simulink model "x64_32s_001"  (Simulink 7.4) (continued)
== system x64_32s_001 == (continued)
      mask CallbackString:
      | |||map_tail = get_param(gcb, 'map_tail');
      | set_param_state(gcb, 'LargerFFTSize', map_tail);
      | set_param_state(gcb, 'StartStage', map_tail);|||||||||fft_callback_arch(gcb);|||specify_mult = get_param(gcb, 'specify_mult');
      | set_param_state(gcb, 'mult_spec', specify_mult);||hardcode_shifts = get_param(gcb, 'hardcode_shifts');
      | set_param_state(gcb, 'shift_schedule', hardcode_shifts);||fft_callback_dsp48_adders(gcb);
      mask TabNameString: Basic,Basic,Basic,Basic,Basic,Basic,Latency,Latency,Latency,Latency,Implementation,Implementation,Implementation,Implementation,Implementation,Implementation,Implementation,Implementation,Implementation,Implementation
  fft_direct2 : Subsystem  AncestorBlock=casper_library_ffts/fft_direct  mask(fft_direct){FFTSize=3, input_bit_width=18, coeff_bit_width=18, map_tail=off, LargerFFTSize=5, StartStage=4, add_latency=2, mult_latency=2, bram_latency=2, conv_latency=2, quantization=Round  (unbiased: +/- Inf), overflow=Saturate, arch=Virtex5, opt_target=logic, coeffs_bit_limit=8, specify_mult=off, mult_spec=[2                                      2], hardcode_shifts=off, shift_schedule=[1                                      1], dsp48_adders=on}
      mask prompts: FFTSize "Size of FFT: (2^? pts)"; input_bit_width "Input/Output Bit Width"; coeff_bit_width "Coefficient Bit Width"; map_tail "Map Tail of Larger FFT"; LargerFFTSize "Size of Larger FFT (2^?)"; StartStage "Start Stage of Map Tail"; add_latency "Add Latency"; mult_latency "Mult Latency"; bram_latency "BRAM Latency"; conv_latency "Convert latency"; quantization "Quantization Behavior"; overflow "Overflow Behavior"; arch "Architecture"; opt_target "Use less"; coeffs_bit_limit "Number bits above which to store stage's coefficients in BRAM (2^? bits)"; specify_mult "Specify multiplier use (behavioural HDL if not specified)"; mult_spec "Multiplier specification (0=core, 1=behavioural HDL, 2=embedded) (left=1st stage)"; hardcode_shifts "Hardcode shift schedule"; shift_schedule "Shift schedule"; dsp48_adders "DSP48 adders in butterfly"
      mask help:
      | FFT internal block.
      |
      | Implements an N-point FFT as the classic butterfly structure,
      | with N inputs and N outputs.
      mask init: same as x64_32s_001/fft_direct1
      settings: AttributesFormatString=|
      | Virtex5
      | stages [4  5  6] of 3
      | [18,18]
      | Round  (unbiased: +/- Inf)
      | Saturate
      mask StyleString: edit,edit,edit,checkbox,edit,edit,edit,edit,edit,edit,popup(Truncate|Round  (unbiased: +/- Inf)|Round  (unbiased: Even Values)),popup(Wrap|Saturate|Error),popup(Virtex2Pro|Virtex5),popup(logic|multipliers),edit,checkbox,edit,checkbox,edit,checkbox
      mask CallbackString:
      | |||map_tail = get_param(gcb, 'map_tail');
      | set_param_state(gcb, 'LargerFFTSize', map_tail);
      | set_param_state(gcb, 'StartStage', map_tail);|||||||||fft_callback_arch(gcb);|||specify_mult = get_param(gcb, 'specify_mult');
      | set_param_state(gcb, 'mult_spec', specify_mult);||hardcode_shifts = get_param(gcb, 'hardcode_shifts');
      | set_param_state(gcb, 'shift_schedule', hardcode_shifts);||fft_callback_dsp48_adders(gcb);
      mask TabNameString: Basic,Basic,Basic,Basic,Basic,Basic,Latency,Latency,Latency,Latency,Implementation,Implementation,Implementation,Implementation,Implementation,Implementation,Implementation,Implementation,Implementation,Implementation
  inst_seng_id0 : Subsystem  Tag=xps:sw_reg  mask{io_dir=To Processor, arith_type=Unsigned, bitwidth=32, bin_pt=0, sample_period=1, gw_name=x64_32s_001_inst_seng_id0_user_data_in}
      mask prompts: io_dir "I/O direction"; arith_type "Data Type"; bitwidth "Data bitwidth"; bin_pt "Data binary point"; sample_period "Sample period"; gw_name "Mask Parameter 6:"
      mask init:
      | register_mask;
      mask StyleString: popup(From Processor|To Processor),popup(Unsigned|Signed  (2's comp)),edit,edit,edit,edit
  mcnt_reg1 : xbsIndex_r4/Register  init=0  rst=off  en=on  dbl_ovrd=off  block_type=register
      settings: block_version=11.4
  mini_vacc : Subsystem
  mini_vacc_mux1 : Subsystem  mask{vec_len=3}
      mask prompts: vec_len "vector length (2^?)"
  payload_cnt1 : S-Function  LoadFcn=xlBlockLoadCallback(gcbh);  FunctionName=sysgen  Parameters=ptable_  EnableBusSupport=off  mask(Xilinx Counter Block){infoedit=Hardware notes: Free running counters are the least expensive in hardware.  A count limited counter is implemented by combining a counter with a comparator., cnt_type_sgadvanced=, cnt_type=Free Running, cnt_to=0, operation_sgadvanced=, operation=Up, start_count=0, cnt_by_val=1, arith_type_sgadvanced=, arith_type=Unsigned, n_bits=32, bin_pt=0, load_pin_sgadvanced=, load_pin=off, rst_sgadvanced=, rst=on, en_sgadvanced=, en=on, explicit_period_sgadvanced=, explicit_period=off, period=1, dbl_ovrd_sgadvanced=, dbl_ovrd=off, use_behavioral_HDL_sgadvanced=, use_behavioral_HDL=off, implementation_sgadvanced=, implementation=Fabric, xl_use_area_sgadvanced=, xl_use_area=off, xl_area=[17 32 0 32 0 0 0], use_rpm=on, xlcconfig=, has_advanced_control=0, sggui_pos=20,20,348,618, block_type=counter, block_version=10.1.3, sg_icon_stat=30,35,2,1,white,blue,0,d605779c,right,, sg_mask_display=|, sg_list_contents=, sg_blockgui_xml=}
      mask sg_mask_display=|
      | fprintf('','COMMENT: begin icon graphics');
      | patch([0 1 1 0 ],[0 0 1 1 ],[0.77 0.82 0.91]);
      | patch([0.233333 0.0666667 0.3 0.0666667 0.233333 0.5 0.566667 0.633333 0.9 0.666667 0.466667 0.3 0.5 0.3 0.466667 0.666667 0.9 0.633333 0.566667 0.5 0.233333 ],[0.171429 0.314286 0.514286 0.714286 0.857143 0.857143 0.8 0.857143 0.857143 0.657143 0.828571 0.685714 0.514286 0.342857 0.2 0.371429 0.171429 0.171429 0.228571 0.171429 0.171429 ],[0.98 0.96 0.92]);
      | plot([0 1 1 0 0 ],[0 0 1 1 0 ]);
      | fprintf('','COMMENT: end icon graphics');
      | fprintf('','COMMENT: begin icon text');
      | color('black');port_label('input',1,'rst');
      | color('black');port_label('input',2,'en');
      | color('black');port_label('output',1,'out');
      | fprintf('','COMMENT: end icon text');
      mask prompts: infoedit ""; cnt_type_sgadvanced ""; cnt_type "Counter type"; cnt_to "Count to value"; operation_sgadvanced ""; operation "Count direction"; start_count "Initial value"; cnt_by_val "Step"; arith_type_sgadvanced ""; arith_type "Output type"; n_bits "Number of bits"; bin_pt "Binary point"; load_pin_sgadvanced ""; load_pin "Provide load port"; rst_sgadvanced ""; rst "Provide synchronous reset port"; en_sgadvanced ""; en "Provide enable port"; explicit_period_sgadvanced ""; explicit_period "Sample period source"; period "Explicit period"; dbl_ovrd_sgadvanced ""; dbl_ovrd "Override with doubles"; use_behavioral_HDL_sgadvanced ""; use_behavioral_HDL "Use behavioral HDL (otherwise use core)"; implementation_sgadvanced ""; implementation "Implement using"; xl_use_area_sgadvanced ""; xl_use_area "Define FPGA area for resource estimation"; xl_area "FPGA area [slices, FFs, BRAMs, LUTs, IOBs, emb. mults, TBUFs]"; use_rpm ""; xlcconfig ""; has_advanced_control ""; sggui_pos ""; block_type ""; block_version ""; sg_icon_stat ""; sg_mask_display ""; sg_list_contents ""; sg_blockgui_xml ""
      mask help: Hardware notes: Free running counters are the least expensive in hardware. A count limited counter is implemented by combining a counter with a comparator.
      mask init:
      | try
      |   tmp_gcb = gcb;
      |   tmp_gcbh = gcbh;
      |   if (strcmp('SysGenIndex',get_param(bdroot(tmp_gcbh),'tag')) && ~isempty(regexp(bdroot(tmp_gcb), '^xbs', 'once')))
      |     return;
      |   end;
      |   xlMungeMaskParams;
      |   block_config = 'sysgen_blockset:Counter_config';
      | 
      |   block_type='counter';
      | 
      |   serialized_declarations = '{''arith_type''=>''Int'',''bin_pt''=>''Int'',''cnt_by_val''=>''Double'',''cnt_to''=>''Double'',''cnt_type''=>''Int'',''en''=>''Int'',''explicit_period''=>''Int'',''implementation''=>''String'',''load_pin''=>''Int'',''n_bits''=>''Int'',''operation''=>''Int'',''period''=>''Double'',''rst''=>''Int'',''start_count''=>''Double'',''use_behavioral_HDL''=>''Int'',''use_rpm''=>''String'',''xlcconfig''=>''String'',''block_type''=>''String''}';
      |   ptable_ = xlblockprep(get_param(tmp_gcb, 'MaskWSVariables'));
      | catch
      |   global dbgsysgen;
      |   if(~isempty(dbgsysgen) && dbgsysgen)
      |     e = regexprep(lasterr, '\n', '\nError: ');
      |     disp(['Error: While running MaskInit code on block ' tmp_gcb ': ' e]);
      |     error(e);
      |   end
      | end
      settings: CopyFcn=xlBlockMoveCallback(gcbh);  DeleteFcn=xlDestroyGui(gcbh);  ModelCloseFcn=xlDestroyGui(gcbh);  PreSaveFcn=xlBlockPreSaveCallback(gcbh);  PostSaveFcn=xlBlockPostSaveCallback(gcbh);  DestroyFcn=xlDestroyGui(gcbh);  OpenFcn=xlOpenGui(gcbh, 'counter_gui.xml', @counterenablement, -1)  CloseFcn=xlDestroyGui(gcbh);  MoveFcn=xlBlockMoveCallback(gcbh);
      mask Help: eval('');xlDoc('-book','sysgen','-topic','Counter');
      mask StyleString: edit,edit,popup(Free Running|Count Limited),edit,edit,popup(Up|Down|Up/Down),edit,edit,edit,popup(Signed  (2's comp)|Unsigned),edit,edit,edit,checkbox,edit,checkbox,edit,checkbox,edit,popup(on|off),edit,edit,checkbox,edit,checkbox,edit,popup(Fabric|DSP48),edit,checkbox,edit,edit,edit,edit,edit,edit,edit,edit,edit,edit,edit
      mask Display:
      | fprintf('','COMMENT: begin icon graphics');
      | patch([0 1 1 0 ],[0 0 1 1 ],[0.77 0.82 0.91]);
      | patch([0.233333 0.0666667 0.3 0.0666667 0.233333 0.5 0.566667 0.633333 0.9 0.666667 0.466667 0.3 0.5 0.3 0.466667 0.666667 0.9 0.633333 0.566667 0.5 0.233333 ],[0.171429 0.314286 0.514286 0.714286 0.857143 0.857143 0.8 0.857143 0.857143 0.657143 0.828571 0.685714 0.514286 0.342857 0.2 0.371429 0.171429 0.171429 0.228571 0.171429 0.171429 ],[0.98 0.96 0.92]);
      | plot([0 1 1 0 0 ],[0 0 1 1 0 ]);
      | fprintf('','COMMENT: end icon graphics');
      | fprintf('','COMMENT: begin icon text');
      | color('black');port_label('input',1,'rst');
      | color('black');port_label('input',2,'en');
      | color('black');port_label('output',1,'out');
      | fprintf('','COMMENT: end icon text');
  payload_cnt2 : S-Function  LoadFcn=xlBlockLoadCallback(gcbh);  FunctionName=sysgen  Parameters=ptable_  EnableBusSupport=off  mask(Xilinx Counter Block){infoedit=Hardware notes: Free running counters are the least expensive in hardware.  A count limited counter is implemented by combining a counter with a comparator., cnt_type_sgadvanced=, cnt_type=Free Running, cnt_to=0, operation_sgadvanced=, operation=Up, start_count=0, cnt_by_val=1, arith_type_sgadvanced=, arith_type=Unsigned, n_bits=32, bin_pt=0, load_pin_sgadvanced=, load_pin=off, rst_sgadvanced=, rst=on, en_sgadvanced=, en=on, explicit_period_sgadvanced=, explicit_period=off, period=1, dbl_ovrd_sgadvanced=, dbl_ovrd=off, use_behavioral_HDL_sgadvanced=, use_behavioral_HDL=off, implementation_sgadvanced=, implementation=Fabric, xl_use_area_sgadvanced=, xl_use_area=off, xl_area=[17 32 0 32 0 0 0], use_rpm=on, xlcconfig=, has_advanced_control=0, sggui_pos=20,20,348,618, block_type=counter, block_version=10.1.3, sg_icon_stat=30,35,2,1,white,blue,0,d605779c,right,, sg_mask_display=|, sg_list_contents=, sg_blockgui_xml=}
      mask sg_mask_display=|
      | fprintf('','COMMENT: begin icon graphics');
      | patch([0 1 1 0 ],[0 0 1 1 ],[0.77 0.82 0.91]);
      | patch([0.233333 0.0666667 0.3 0.0666667 0.233333 0.5 0.566667 0.633333 0.9 0.666667 0.466667 0.3 0.5 0.3 0.466667 0.666667 0.9 0.633333 0.566667 0.5 0.233333 ],[0.171429 0.314286 0.514286 0.714286 0.857143 0.857143 0.8 0.857143 0.857143 0.657143 0.828571 0.685714 0.514286 0.342857 0.2 0.371429 0.171429 0.171429 0.228571 0.171429 0.171429 ],[0.98 0.96 0.92]);
      | plot([0 1 1 0 0 ],[0 0 1 1 0 ]);
      | fprintf('','COMMENT: end icon graphics');
      | fprintf('','COMMENT: begin icon text');
      | color('black');port_label('input',1,'rst');
      | color('black');port_label('input',2,'en');
      | color('black');port_label('output',1,'out');
      | fprintf('','COMMENT: end icon text');
      mask prompts: infoedit ""; cnt_type_sgadvanced ""; cnt_type "Counter type"; cnt_to "Count to value"; operation_sgadvanced ""; operation "Count direction"; start_count "Initial value"; cnt_by_val "Step"; arith_type_sgadvanced ""; arith_type "Output type"; n_bits "Number of bits"; bin_pt "Binary point"; load_pin_sgadvanced ""; load_pin "Provide load port"; rst_sgadvanced ""; rst "Provide synchronous reset port"; en_sgadvanced ""; en "Provide enable port"; explicit_period_sgadvanced ""; explicit_period "Sample period source"; period "Explicit period"; dbl_ovrd_sgadvanced ""; dbl_ovrd "Override with doubles"; use_behavioral_HDL_sgadvanced ""; use_behavioral_HDL "Use behavioral HDL (otherwise use core)"; implementation_sgadvanced ""; implementation "Implement using"; xl_use_area_sgadvanced ""; xl_use_area "Define FPGA area for resource estimation"; xl_area "FPGA area [slices, FFs, BRAMs, LUTs, IOBs, emb. mults, TBUFs]"; use_rpm ""; xlcconfig ""; has_advanced_control ""; sggui_pos ""; block_type ""; block_version ""; sg_icon_stat ""; sg_mask_display ""; sg_list_contents ""; sg_blockgui_xml ""
      mask help: Hardware notes: Free running counters are the least expensive in hardware. A count limited counter is implemented by combining a counter with a comparator.
      mask init: same as x64_32s_001/payload_cnt1
      settings: CopyFcn=xlBlockMoveCallback(gcbh);  DeleteFcn=xlDestroyGui(gcbh);  ModelCloseFcn=xlDestroyGui(gcbh);  PreSaveFcn=xlBlockPreSaveCallback(gcbh);  PostSaveFcn=xlBlockPostSaveCallback(gcbh);  DestroyFcn=xlDestroyGui(gcbh);  OpenFcn=xlOpenGui(gcbh, 'counter_gui.xml', @counterenablement, -1)  CloseFcn=xlDestroyGui(gcbh);  MoveFcn=xlBlockMoveCallback(gcbh);
      mask Help: eval('');xlDoc('-book','sysgen','-topic','Counter');
      mask StyleString: edit,edit,popup(Free Running|Count Limited),edit,edit,popup(Up|Down|Up/Down),edit,edit,edit,popup(Signed  (2's comp)|Unsigned),edit,edit,edit,checkbox,edit,checkbox,edit,checkbox,edit,popup(on|off),edit,edit,checkbox,edit,checkbox,edit,popup(Fabric|DSP48),edit,checkbox,edit,edit,edit,edit,edit,edit,edit,edit,edit,edit,edit
      mask Display:
      | fprintf('','COMMENT: begin icon graphics');
      | patch([0 1 1 0 ],[0 0 1 1 ],[0.77 0.82 0.91]);
      | patch([0.233333 0.0666667 0.3 0.0666667 0.233333 0.5 0.566667 0.633333 0.9 0.666667 0.466667 0.3 0.5 0.3 0.466667 0.666667 0.9 0.633333 0.566667 0.5 0.233333 ],[0.171429 0.314286 0.514286 0.714286 0.857143 0.857143 0.8 0.857143 0.857143 0.657143 0.828571 0.685714 0.514286 0.342857 0.2 0.371429 0.171429 0.171429 0.228571 0.171429 0.171429 ],[0.98 0.96 0.92]);
      | plot([0 1 1 0 0 ],[0 0 1 1 0 ]);
      | fprintf('','COMMENT: end icon graphics');
      | fprintf('','COMMENT: begin icon text');
      | color('black');port_label('input',1,'rst');
      | color('black');port_label('input',2,'en');
      | color('black');port_label('output',1,'out');
      | fprintf('','COMMENT: end icon text');
  payload_cnt3 : S-Function  LoadFcn=xlBlockLoadCallback(gcbh);  FunctionName=sysgen  Parameters=ptable_  EnableBusSupport=off  mask(Xilinx Counter Block){infoedit=Hardware notes: Free running counters are the least expensive in hardware.  A count limited counter is implemented by combining a counter with a comparator., cnt_type_sgadvanced=, cnt_type=Free Running, cnt_to=0, operation_sgadvanced=, operation=Up, start_count=0, cnt_by_val=1, arith_type_sgadvanced=, arith_type=Unsigned, n_bits=32, bin_pt=0, load_pin_sgadvanced=, load_pin=off, rst_sgadvanced=, rst=on, en_sgadvanced=, en=on, explicit_period_sgadvanced=, explicit_period=off, period=1, dbl_ovrd_sgadvanced=, dbl_ovrd=off, use_behavioral_HDL_sgadvanced=, use_behavioral_HDL=off, implementation_sgadvanced=, implementation=Fabric, xl_use_area_sgadvanced=, xl_use_area=off, xl_area=[17 32 0 32 0 0 0], use_rpm=on, xlcconfig=, has_advanced_control=0, sggui_pos=20,20,348,618, block_type=counter, block_version=10.1.3, sg_icon_stat=30,35,2,1,white,blue,0,d605779c,right,, sg_mask_display=|, sg_list_contents=, sg_blockgui_xml=}
      mask sg_mask_display=|
      | fprintf('','COMMENT: begin icon graphics');
      | patch([0 1 1 0 ],[0 0 1 1 ],[0.77 0.82 0.91]);
      | patch([0.233333 0.0666667 0.3 0.0666667 0.233333 0.5 0.566667 0.633333 0.9 0.666667 0.466667 0.3 0.5 0.3 0.466667 0.666667 0.9 0.633333 0.566667 0.5 0.233333 ],[0.171429 0.314286 0.514286 0.714286 0.857143 0.857143 0.8 0.857143 0.857143 0.657143 0.828571 0.685714 0.514286 0.342857 0.2 0.371429 0.171429 0.171429 0.228571 0.171429 0.171429 ],[0.98 0.96 0.92]);
      | plot([0 1 1 0 0 ],[0 0 1 1 0 ]);
      | fprintf('','COMMENT: end icon graphics');
      | fprintf('','COMMENT: begin icon text');
      | color('black');port_label('input',1,'rst');
      | color('black');port_label('input',2,'en');
      | color('black');port_label('output',1,'out');
      | fprintf('','COMMENT: end icon text');
      mask prompts: infoedit ""; cnt_type_sgadvanced ""; cnt_type "Counter type"; cnt_to "Count to value"; operation_sgadvanced ""; operation "Count direction"; start_count "Initial value"; cnt_by_val "Step"; arith_type_sgadvanced ""; arith_type "Output type"; n_bits "Number of bits"; bin_pt "Binary point"; load_pin_sgadvanced ""; load_pin "Provide load port"; rst_sgadvanced ""; rst "Provide synchronous reset port"; en_sgadvanced ""; en "Provide enable port"; explicit_period_sgadvanced ""; explicit_period "Sample period source"; period "Explicit period"; dbl_ovrd_sgadvanced ""; dbl_ovrd "Override with doubles"; use_behavioral_HDL_sgadvanced ""; use_behavioral_HDL "Use behavioral HDL (otherwise use core)"; implementation_sgadvanced ""; implementation "Implement using"; xl_use_area_sgadvanced ""; xl_use_area "Define FPGA area for resource estimation"; xl_area "FPGA area [slices, FFs, BRAMs, LUTs, IOBs, emb. mults, TBUFs]"; use_rpm ""; xlcconfig ""; has_advanced_control ""; sggui_pos ""; block_type ""; block_version ""; sg_icon_stat ""; sg_mask_display ""; sg_list_contents ""; sg_blockgui_xml ""
      mask help: Hardware notes: Free running counters are the least expensive in hardware. A count limited counter is implemented by combining a counter with a comparator.
      mask init: same as x64_32s_001/payload_cnt1
      settings: CopyFcn=xlBlockMoveCallback(gcbh);  DeleteFcn=xlDestroyGui(gcbh);  ModelCloseFcn=xlDestroyGui(gcbh);  PreSaveFcn=xlBlockPreSaveCallback(gcbh);  PostSaveFcn=xlBlockPostSaveCallback(gcbh);  DestroyFcn=xlDestroyGui(gcbh);  OpenFcn=xlOpenGui(gcbh, 'counter_gui.xml', @counterenablement, -1)  CloseFcn=xlDestroyGui(gcbh);  MoveFcn=xlBlockMoveCallback(gcbh);
      mask Help: eval('');xlDoc('-book','sysgen','-topic','Counter');
      mask StyleString: edit,edit,popup(Free Running|Count Limited),edit,edit,popup(Up|Down|Up/Down),edit,edit,edit,popup(Signed  (2's comp)|Unsigned),edit,edit,edit,checkbox,edit,checkbox,edit,checkbox,edit,popup(on|off),edit,edit,checkbox,edit,checkbox,edit,popup(Fabric|DSP48),edit,checkbox,edit,edit,edit,edit,edit,edit,edit,edit,edit,edit,edit
      mask Display:
      | fprintf('','COMMENT: begin icon graphics');
      | patch([0 1 1 0 ],[0 0 1 1 ],[0.77 0.82 0.91]);
      | patch([0.233333 0.0666667 0.3 0.0666667 0.233333 0.5 0.566667 0.633333 0.9 0.666667 0.466667 0.3 0.5 0.3 0.466667 0.666667 0.9 0.633333 0.566667 0.5 0.233333 ],[0.171429 0.314286 0.514286 0.714286 0.857143 0.857143 0.8 0.857143 0.857143 0.657143 0.828571 0.685714 0.514286 0.342857 0.2 0.371429 0.171429 0.171429 0.228571 0.171429 0.171429 ],[0.98 0.96 0.92]);
      | plot([0 1 1 0 0 ],[0 0 1 1 0 ]);
      | fprintf('','COMMENT: end icon graphics');
      | fprintf('','COMMENT: begin icon text');
      | color('black');port_label('input',1,'rst');
      | color('black');port_label('input',2,'en');
      | color('black');port_label('output',1,'out');
      | fprintf('','COMMENT: end icon text');
  pow_sim_mux : Subsystem
  pow_sim_mux1 : Subsystem
  pow_sim_mux10 : Subsystem
  pow_sim_mux11 : Subsystem
  pow_sim_mux12 : Subsystem
  pow_sim_mux13 : Subsystem
  pow_sim_mux14 : Subsystem
  pow_sim_mux15 : Subsystem
  pow_sim_mux2 : Subsystem
  pow_sim_mux3 : Subsystem
  pow_sim_mux4 : Subsystem
  pow_sim_mux5 : Subsystem
  pow_sim_mux6 : Subsystem
  pow_sim_mux7 : Subsystem
  pow_sim_mux8 : Subsystem
  pow_sim_mux9 : Subsystem
  qdr_in_cnt : Subsystem  Tag=xps:sw_reg  mask{io_dir=To Processor, arith_type=Unsigned, bitwidth=32, bin_pt=0, sample_period=1, gw_name=x64_32s_001_qdr_in_cnt_user_data_in}
      mask prompts: io_dir "I/O direction"; arith_type "Data Type"; bitwidth "Data bitwidth"; bin_pt "Data binary point"; sample_period "Sample period"; gw_name "Mask Parameter 6:"
      mask init:
      | register_mask;
      mask StyleString: popup(From Processor|To Processor),popup(Unsigned|Signed  (2's comp)),edit,edit,edit,edit
  qdr_vacc : Subsystem  AncestorBlock=casper_library_accumulators/qdr_vacc  mask{vector_len=1024*32*4}
      mask prompts: vector_len "Vector length??(# cycles)"
      mask help:
      | A vector accumulator for medium length vectors designed for ROACH's QDR memory.
      |
      | Originally designed for the packetised correlator, this block accepts input data of type Fix_32_0. OOB_data_in provides an extra 4 bits that are accumulated seperately (originally designed to keep track of how many "bad" values in a vector were accumulated).
      |
      | Minimum vector length is 2 * burst_length.
      | Minimum burst length is 10 (recommended default is 2^4=16).
      |
      | The vector length does not need to be a multiple of the burst length.
      mask init:
      | vector_bits = ceil(log2(vector_len));
      | burst_bits = 4;
      | burst_len = 2^burst_bits;
      | 
      | fmtstr = sprintf('Vector Length: %d', vector_len);
      | set_param(gcb, 'AttributesFormatString', fmtstr);
      settings: AttributesFormatString=Vector Length: 131072
  snap_vacc0 : Subsystem  AncestorBlock=casper_library/Scopes/snap  mask(snap){nsamples=11}
      mask prompts: nsamples "No. of samples(2^?)"
      mask help:
      | The <i>snap</i> block provides a packaged solution to capturing data from
      | the FPGA fabric and making it accessible from the CPU.  The block comes in
      | 2 flavors: <i>snap</i> (which captures to a 32 bit wide Shared BRAM), and
      | <i>snap64</i> (which captures to 2x32 bit wide Shared BRAMs to effect a
      | 64 bit capture).
      mask Help: eval('xlWeb([getenv(''MLIB_ROOT''), ''\doc\htmlblocks\sys_snap\sys_snap.html''])')
  square_transposer : Subsystem  AncestorBlock=casper_library/Reorder/square_transposer  mask(square_transposer){n_inputs=3}
      mask prompts: n_inputs "Number of inputs (2^?):"
      mask init:
      | square_transposer_init(gcb,...
      |     'n_inputs', n_inputs);
      settings: AttributesFormatString=n_inputs=3
      mask Help: eval('xlWeb(''http://casper.berkeley.edu/wiki/Square_transposer'')')
  status : Subsystem  Tag=xps:sw_reg  mask{io_dir=To Processor, arith_type=Unsigned, bitwidth=32, bin_pt=0, sample_period=1, gw_name=x64_32s_001_status_user_data_in}
      mask prompts: io_dir "I/O direction"; arith_type "Data Type"; bitwidth "Data bitwidth"; bin_pt "Data binary point"; sample_period "Sample period"; gw_name "Mask Parameter 6:"
      mask init:
      | register_mask;
      mask StyleString: popup(From Processor|To Processor),popup(Unsigned|Signed  (2's comp)),edit,edit,edit,edit
  sync_gen : Subsystem  mask{nChans=10, nXeng=0}
      mask prompts: nChans "Number of channels (total) (2^?)"; nXeng "Number of X engines (2^?)"
  uncram1 : Subsystem  AncestorBlock=gavrt_library/uncram  mask(uncram){num_slice=4, slice_width=8, bin_pt=0, arith_type=0}
      mask prompts: num_slice "Number of slices"; slice_width "Slice Width"; bin_pt "Output Binary Point"; arith_type "Output Arithmetic Type (0=Unsigned, 1=Signed)"
      mask help: Takes a concatenated input and slices it up into even pieces and reinterprets them as signed fixed point numbers with a given binary point.
      mask init:
      | uncram_init(gcb, ...
      |     'num_slice', num_slice, ...
      |     'slice_width', slice_width, ...
      |     'bin_pt', bin_pt, ...
      |     'arith_type', arith_type);
      settings: AttributesFormatString=UFix_8_0
  vacc_cnt0 : Subsystem  Tag=xps:sw_reg  mask{io_dir=To Processor, arith_type=Unsigned, bitwidth=32, bin_pt=0, sample_period=1, latency=0}
      mask prompts: io_dir "I/O direction"; arith_type "Data Type"; bitwidth "Data bitwidth"; bin_pt "Data binary point"; sample_period "Sample period"
      mask init:
      | gw_name = clear_name(gcb);
      | config.source = str2func('software_register_init');
      | config.toplevel = gcb;
      | xBlock( config, {io_dir, arith_type, bitwidth, bin_pt, ... 
      |     sample_period, gw_name, latency});
      mask StyleString: popup(From Processor|To Processor),popup(Unsigned|Signed  (2's comp)),edit,edit,edit,edit
  vacc_err_cnt0 : Subsystem  Tag=xps:sw_reg  mask{io_dir=To Processor, arith_type=Unsigned, bitwidth=32, bin_pt=0, sample_period=1, latency=0}
      mask prompts: io_dir "I/O direction"; arith_type "Data Type"; bitwidth "Data bitwidth"; bin_pt "Data binary point"; sample_period "Sample period"
      mask init:
      | gw_name = clear_name(gcb);
      | config.source = str2func('software_register_init');
      | config.toplevel = gcb;
      | xBlock( config, {io_dir, arith_type, bitwidth, bin_pt, ... 
      |     sample_period, gw_name, latency});
      mask StyleString: popup(From Processor|To Processor),popup(Unsigned|Signed  (2's comp)),edit,edit,edit,edit
  vacc_mcnt_h0 : Subsystem  Tag=xps:sw_reg  mask{io_dir=To Processor, arith_type=Unsigned, bitwidth=32, bin_pt=0, sample_period=1, latency=0}
      mask prompts: io_dir "I/O direction"; arith_type "Data Type"; bitwidth "Data bitwidth"; bin_pt "Data binary point"; sample_period "Sample period"
      mask init:
      | gw_name = clear_name(gcb);
      | config.source = str2func('software_register_init');
      | config.toplevel = gcb;
      | xBlock( config, {io_dir, arith_type, bitwidth, bin_pt, ... 
      |     sample_period, gw_name, latency});
      mask StyleString: popup(From Processor|To Processor),popup(Unsigned|Signed  (2's comp)),edit,edit,edit,edit
  vacc_mcnt_l0 : Subsystem  Tag=xps:sw_reg  mask{io_dir=To Processor, arith_type=Unsigned, bitwidth=32, bin_pt=0, sample_period=1, latency=0}
      mask prompts: io_dir "I/O direction"; arith_type "Data Type"; bitwidth "Data bitwidth"; bin_pt "Data binary point"; sample_period "Sample period"
      mask init:
      | gw_name = clear_name(gcb);
      | config.source = str2func('software_register_init');
      | config.toplevel = gcb;
      | xBlock( config, {io_dir, arith_type, bitwidth, bin_pt, ... 
      |     sample_period, gw_name, latency});
      mask StyleString: popup(From Processor|To Processor),popup(Unsigned|Signed  (2's comp)),edit,edit,edit,edit
  vacc_monitor : Subsystem
  vacc_monitor1 : Subsystem
  vacc_monitor2 : Subsystem
  vacc_sim_mux : Subsystem
  x_window : Subsystem
  xaui_TVG : Subsystem
  xaui_buf0 : Subsystem
  xaui_cnt0 : Subsystem  Tag=xps:sw_reg  mask{io_dir=To Processor, arith_type=Unsigned, bitwidth=32, bin_pt=0, sample_period=1, gw_name=x64_32s_001_xaui_cnt0_user_data_in}
      mask prompts: io_dir "I/O direction"; arith_type "Data Type"; bitwidth "Data bitwidth"; bin_pt "Data binary point"; sample_period "Sample period"; gw_name "Mask Parameter 6:"
      mask init:
      | register_mask;
      mask StyleString: popup(From Processor|To Processor),popup(Unsigned|Signed  (2's comp)),edit,edit,edit,edit
  xaui_error_cnt0 : Subsystem  Tag=xps:sw_reg  mask{io_dir=To Processor, arith_type=Unsigned, bitwidth=32, bin_pt=0, sample_period=1, gw_name=x64_32s_001_xaui_error_cnt0_user_data_in}
      mask prompts: io_dir "I/O direction"; arith_type "Data Type"; bitwidth "Data bitwidth"; bin_pt "Data binary point"; sample_period "Sample period"; gw_name "Mask Parameter 6:"
      mask init:
      | register_mask;
      mask StyleString: popup(From Processor|To Processor),popup(Unsigned|Signed  (2's comp)),edit,edit,edit,edit
  xaui_pack0 : Subsystem  mask{payload_len=2^5, xeng_bits=0, skip_xeng_bits=0, skip_ant_bits=4}
      mask prompts: payload_len "Payload Length (in 64 bit words)"; xeng_bits "Number of X engines (2^?)"; skip_xeng_bits "X engines per IP address (2^?)"; skip_ant_bits "Antennas per XAUI link (2^?)"
      mask help: Packetizes data coming in over a XAUI interface. A packet consists of a 64 bit header (48 bits of "mcnt" and 16 bits of antenna), followed by 64 x "payload" bits of payload. "Mcnt" (master count) is a counter which keeps track of how many packets have been transmitted since the last "mrst".
      mask init:
      | packet_len = payload_len + 1;
      | packet_bits = ceil(log2(packet_len));
      | payload_bits = ceil(log2(payload_len));
  xaui_sim_mux2 : Subsystem  mask{xaui_lat=2, start_val=1}
      mask prompts: xaui_lat "simulated XAUI latency"; start_val "count start value"
  xauibuf_out_cnt : Subsystem  Tag=xps:sw_reg  mask{io_dir=To Processor, arith_type=Unsigned, bitwidth=32, bin_pt=0, sample_period=1, gw_name=x64_32s_001_xauibuf_out_cnt_user_data_in}
      mask prompts: io_dir "I/O direction"; arith_type "Data Type"; bitwidth "Data bitwidth"; bin_pt "Data binary point"; sample_period "Sample period"; gw_name "Mask Parameter 6:"
      mask init:
      | register_mask;
      mask StyleString: popup(From Processor|To Processor),popup(Unsigned|Signed  (2's comp)),edit,edit,edit,edit
  xauibuf_window_cnt : Subsystem  Tag=xps:sw_reg  mask{io_dir=To Processor, arith_type=Unsigned, bitwidth=32, bin_pt=0, sample_period=1, gw_name=x64_32s_001_xauibuf_window_cnt_user_data_in}
      mask prompts: io_dir "I/O direction"; arith_type "Data Type"; bitwidth "Data bitwidth"; bin_pt "Data binary point"; sample_period "Sample period"; gw_name "Mask Parameter 6:"
      mask init:
      | register_mask;
      mask StyleString: popup(From Processor|To Processor),popup(Unsigned|Signed  (2's comp)),edit,edit,edit,edit
  y_window : Subsystem
  zero_mask : Subsystem
  zero_mask1 : Subsystem
  wires:
  Constant7/1 -> XAUI/1
  [xaui_rcv_rst] -> XAUI/2
  XAUI/1 -> xaui_sim_mux2/1(rx_data)
  XAUI/2 -> xaui_sim_mux2/2(rx_oob)
  XAUI/3 -> xaui_sim_mux2/3(rx_empty)
  XAUI/4 -> xaui_sim_mux2/4(rx_valid)
  XAUI/5 -> xaui_sim_mux2/5(rx_linkdown)
  XAUI/6 -> xaui_sim_mux2/6(tx_full)
  XAUI/7 -> xaui_sim_mux2/7(rx_almost_full)
  xaui_sim_mux2/7(af) -> Terminator21/1
  Constant20/1 -> ctrl_sw/1(sim_reg_in)
  ctrl_sw/1(reg_in) -> Slice/1, Slice1/1, Slice4/1, Slice5/1, Slice7/1, Slice10/1, Slice3/1, Slice2/1, Slice6/1
  Slice/1 -> [x_fft_shift]
  Constant1/1 -> XAUI/3
  Constant2/1 -> XAUI/4
  Constant3/1 -> XAUI/5
  [data_buf] -> uncram1/1(In)
  [x_fft_shift] -> fft_direct2/2(shift)
  Constant4/1 -> fft_direct2/7(in4), fft_direct2/8(in5), fft_direct2/9(in6), fft_direct2/10(in7)
  fft_direct2/1(sync_out) -> [x_fft_sync]
  fft_direct2/2(out0) -> [x0]
  fft_direct2/3(out1) -> [x1]
  fft_direct2/4(out2) -> [x2]
  fft_direct2/5(out3) -> [x3]
  fft_direct2/6(out4) -> [x4]
  fft_direct2/7(out5) -> [x5]
  fft_direct2/8(out6) -> [x6]
  fft_direct2/9(out7) -> [x7]
  fft_direct2/10(of) -> [x_fft_of]
  fft_direct1/1(sync_out) -> [y_fft_sync]
  fft_direct1/18(of) -> [y_fft_of]
  [acc_len] -> qdr_vacc/1(n_acum)
  Constant6/1 -> qdr_vacc/4(oob_data_in)
  [qdr_in_vld] -> qdr_vacc/5(we)
  [qdr_vacc_data] -> Convert3/1
  qdr_vacc/1(new_acc (ASYNC)) -> [new_acc]
  [qdr_sync] -> qdr_vacc/2(sync)
  Slice1/1 -> [y_fft_shift]
  acc_len/1(reg_in) -> [acc_len]
  Constant8/1 -> acc_len/1(sim_reg_in)
  Slice2/1 -> [xaui_rcv_rst]
  Slice3/1 -> [acc_ctr_rst]
  [x_fft_sync] -> square_transposer/1(sync)
  [x0] -> square_transposer/2(In1)
  [x1] -> square_transposer/3(In2)
  [x2] -> square_transposer/4(In3)
  [x3] -> square_transposer/5(In4)
  [x4] -> square_transposer/6(In5)
  [x5] -> square_transposer/7(In6)
  [x6] -> square_transposer/8(In7)
  [x7] -> square_transposer/9(In8)
  square_transposer/1(sync_out) -> [ct_sync_out]
  square_transposer/2(Out1) -> [y0]
  square_transposer/3(Out2) -> [y1]
  square_transposer/4(Out3) -> [y2]
  square_transposer/5(Out4) -> [y3]
  square_transposer/6(Out5) -> [y4]
  square_transposer/7(Out6) -> [y5]
  square_transposer/8(Out7) -> [y6]
  square_transposer/9(Out8) -> [y7]
  Constant9/1 -> fft_direct1/12(in9), fft_direct1/11(in8), fft_direct1/13(in10), fft_direct1/14(in11), fft_direct1/15(in12), fft_direct1/16(in13), fft_direct1/17(in14), fft_direct1/18(in15)
  [y_fft_shift] -> fft_direct1/2(shift)
  fft_direct1/2(out0) -> [Y0]
  fft_direct1/3(out1) -> [Y1]
  fft_direct1/4(out2) -> [Y2]
  fft_direct1/5(out3) -> [Y3]
  fft_direct1/6(out4) -> [Y4]
  fft_direct1/7(out5) -> [Y5]
  fft_direct1/8(out6) -> [Y6]
  fft_direct1/9(out7) -> [Y7]
  fft_direct1/10(out8) -> [Y8]
  fft_direct1/11(out9) -> [Y9]
  fft_direct1/12(out10) -> [Y10]
  fft_direct1/13(out11) -> [Y11]
  fft_direct1/14(out12) -> [Y12]
  fft_direct1/15(out13) -> [Y13]
  fft_direct1/16(out14) -> [Y14]
  fft_direct1/17(out15) -> [Y15]
  [Y0] -> 16_power/1(In1)
  [y_fft_sync] -> 16_power/17(sync)
  [Y1] -> 16_power/2(In2)
  [Y2] -> 16_power/3(In3)
  [Y3] -> 16_power/4(In4)
  [Y4] -> 16_power/5(In5)
  [Y5] -> 16_power/6(In6)
  [Y6] -> 16_power/7(In7)
  [Y7] -> 16_power/8(In8)
  [Y8] -> 16_power/9(In9)
  [Y9] -> 16_power/10(In10)
  [Y10] -> 16_power/11(In11)
  [Y11] -> 16_power/12(In12)
  [Y12] -> 16_power/13(In13)
  [Y13] -> 16_power/14(In14)
  [Y14] -> 16_power/15(In15)
  [Y15] -> 16_power/16(In16)
  16_power/17(sync_out) -> [pow_sync_out], pow_sim_mux/1(sync), pow_sim_mux1/1(sync), pow_sim_mux2/1(sync), pow_sim_mux3/1(sync), pow_sim_mux4/1(sync), pow_sim_mux5/1(sync), pow_sim_mux6/1(sync), pow_sim_mux7/1(sync), pow_sim_mux8/1(sync), pow_sim_mux15/1(sync), pow_sim_mux9/1(sync), pow_sim_mux10/1(sync), pow_sim_mux11/1(sync), pow_sim_mux14/1(sync), pow_sim_mux13/1(sync), pow_sim_mux12/1(sync)
  mini_vacc/1(sync_out) -> [mini_vacc_sync_out]
  [pow_sync_out] -> mini_vacc/1(new_acc)
  [Y0_pow] -> Reinterpret/1
  [Y1_pow] -> Reinterpret1/1
  [Y2_pow] -> Reinterpret2/1
  [Y3_pow] -> Reinterpret3/1
  [Y4_pow] -> Reinterpret4/1
  [Y5_pow] -> Reinterpret5/1
  [Y6_pow] -> Reinterpret6/1
  [Y7_pow] -> Reinterpret7/1
  [Y8_pow] -> Reinterpret8/1
  [Y9_pow] -> Reinterpret9/1
  [Y10_pow] -> Reinterpret10/1
  [Y11_pow] -> Reinterpret11/1
  [Y12_pow] -> Reinterpret12/1
  [Y13_pow] -> Reinterpret13/1
  [Y14_pow] -> Reinterpret14/1
  [Y15_pow] -> Reinterpret15/1
  mini_vacc/3(Out1) -> cram/1(In1)
  mini_vacc/4(Out3) -> cram/2(In2)
  mini_vacc/5(Out5) -> cram/3(In3)
  mini_vacc/6(Out7) -> cram/4(In4)
  mini_vacc/7(Out9) -> cram/5(In5)
  mini_vacc/8(Out11) -> cram/6(In6)
  mini_vacc/9(Out13) -> cram/7(In7)
  mini_vacc/10(Out15) -> cram/8(In8)
  mini_vacc/11(Out17) -> cram/9(In9)
  mini_vacc/12(Out19) -> cram/10(In10)
  mini_vacc/13(Out21) -> cram/11(In11)
  mini_vacc/14(Out23) -> cram/12(In12)
  mini_vacc/15(Out25) -> cram/13(In13)
  mini_vacc/16(Out27) -> cram/14(In14)
  mini_vacc/17(Out29) -> cram/15(In15)
  mini_vacc/18(Out31) -> cram/16(In16)
  cram/1(Out) -> [mini_vacc_out]
  xaui_sim_mux2/1(dout) -> xaui_TVG/1(din)
  xaui_sim_mux2/2(oob) -> xaui_TVG/2(oob)
  xaui_sim_mux2/3(empty) -> xaui_TVG/3(empty)
  xaui_sim_mux2/4(valid) -> xaui_TVG/4(vld)
  xaui_sim_mux2/5(linkdown) -> xaui_TVG/5(ld)
  xaui_sim_mux2/6(full) -> xaui_TVG/6(full)
  xaui_TVG/1(dout) -> [xaui0_data]
  xaui_TVG/2(oob_out) -> [xaui0_oob]
  xaui_TVG/3(empty_out) -> [empty_0]
  xaui_TVG/4(vld_out) -> [xaui0_valid]
  xaui_TVG/5(ld_out) -> [xaui0_link_dn]
  xaui_TVG/6(full_out) -> [full_0]
  [xaui_tvg_en] -> xaui_TVG/7(tvg_en)
  Delay24/1(q) -> xaui_pack0/1(din)
  Delay28/1(q) -> xaui_pack0/2(oob)
  Delay29/1(q) -> xaui_pack0/3(valid_in)
  Delay30/1(q) -> xaui_pack0/4(linkdown_in)
  [xaui0_data] -> Delay24/1(d)
  [xaui0_oob] -> Delay28/1(d)
  [xaui0_valid] -> Delay29/1(d)
  [xaui0_link_dn] -> Delay30/1(d)
  Delay33/1(q) -> [feng_sync]
  Delay34/1(q) -> [xaui_link_down]
  Delay35/1(q) -> [xaui_pkt_err]
  Delay36/1(q) -> [feng_mrst]
  Delay37/1(q) -> [xaui_pkt_data]
  Delay32/1(q) -> [xaui_pkt_valid]
  Delay31/1(q) -> [xaui_pkt_eof]
  xaui_pack0/1(dout) -> Delay37/1(d)
  xaui_pack0/2(valid_out) -> Delay32/1(d)
  xaui_pack0/3(eof) -> Delay31/1(d)
  xaui_pack0/4(discard) -> Delay35/1(d)
  xaui_pack0/5(feng_sync) -> Delay33/1(d)
  xaui_pack0/6(linkdown_out) -> Delay34/1(d)
  xaui_pack0/7(mrst) -> Delay36/1(d)
  xaui_pack0/8(hdr) -> Delay27/1(d)
  xaui_pack0/9(hdr_valid) -> Delay38/1(d)
  Delay27/1(q) -> [xaui_pkt_hdr]
  Delay38/1(q) -> [xaui_hdr_valid]
  [xaui_pkt_hdr] -> xaui_buf0/5(hdr_in)
  xaui_buf0/4(mcnt) -> [buf0_mcnt]
  xaui_buf0/5(ant) -> [buf0_ant]
  xaui_buf0/6(flag) -> [buf0_flag]
  [xaui_pkt_eof] -> xaui_buf0/4(eof_in)
  xaui_buf0/3(eof_out) -> [buf0_eof]
  [xaui_pkt_valid] -> xaui_buf0/2(valid)
  xaui_buf0/2(we) -> [buf0_we]
  xaui_buf0/1(dout) -> [buf0_data]
  [xaui_pkt_data] -> xaui_buf0/1(din)
  [xaui_hdr_valid] -> xaui_buf0/3(hdr_valid)
  Slice4/1 -> Simulation Multiplexer1/2
  Simulation Multiplexer1/1 -> [xaui_tvg_en]
  Constant/1 -> Delay1/1
  Delay1/1 -> Simulation Multiplexer1/1
  Mult/1 -> convert_of/1(din)
  [acc_scale] -> Mult/1
  Delay2/1 -> Delay3/1
  Delay3/1 -> Delay5/1, Register13/2
  Concat5/1 -> status/1(reg_out)
  [x_fft_of] -> Register/1, Register/3
  [y_fft_of] -> Register1/1, Register1/3
  Reinterpret/1 -> mini_vacc/2(In2)
  Reinterpret1/1 -> mini_vacc/3(In4)
  Reinterpret2/1 -> mini_vacc/4(In6)
  Reinterpret3/1 -> mini_vacc/5(In8)
  Reinterpret4/1 -> mini_vacc/6(In10)
  Reinterpret5/1 -> mini_vacc/7(In12)
  Reinterpret6/1 -> mini_vacc/8(In14)
  Reinterpret7/1 -> mini_vacc/9(In16)
  Reinterpret8/1 -> mini_vacc/10(In18)
  Reinterpret9/1 -> mini_vacc/11(In20)
  Reinterpret10/1 -> mini_vacc/12(In22)
  Reinterpret11/1 -> mini_vacc/13(In24)
  Reinterpret12/1 -> mini_vacc/14(In26)
  Reinterpret13/1 -> mini_vacc/15(In28)
  Reinterpret14/1 -> mini_vacc/16(In30)
  Reinterpret15/1 -> mini_vacc/17(In32)
  Register13/1 -> sync_gen/1(mcnt), Delay14/1
  [last_mcnt] -> mcnt_reg1/1
  mcnt_reg1/1 -> Convert2/1
  [new_acc] -> mcnt_reg1/2
  Slice8/1 -> vacc_mcnt_l0/1(reg_out)
  Slice9/1 -> vacc_mcnt_h0/1(reg_out)
  Convert2/1 -> Slice8/1, Slice9/1
  mini_vacc/2(vld) -> [mini_vacc_vld_out]
  mini_vacc_mux1/1(dout) -> Reinterpret16/1
  mini_vacc_mux1/2(sync_out) -> Delay2/1, Gateway Out14/1
  mini_vacc_mux1/3(vld) -> Delay8/1, Gateway Out15/1
  Convert3/1 -> qdr_vacc/3(data_in)
  Delay8/1 -> Delay9/1
  Delay9/1 -> Delay4/1
  Reinterpret16/1 -> Mult/2, Gateway Out13/1
  [mini_vacc_sync_out] -> vacc_sim_mux/1(sync), Gateway Out17/1
  [mini_vacc_out] -> vacc_sim_mux/2(vacc_din), Gateway Out18/1
  [mini_vacc_vld_out] -> vacc_sim_mux/3(vld), Gateway Out19/1
  vacc_sim_mux/1(sync_out) -> mini_vacc_mux1/1(sync), Gateway Out10/1
  vacc_sim_mux/2(dout) -> mini_vacc_mux1/2(din), Gateway Out11/1
  vacc_sim_mux/3(vld_out) -> mini_vacc_mux1/3(vld_in), Gateway Out12/1
  Gateway Out10/1 -> Scope2/1
  Gateway Out11/1 -> Scope2/2
  Gateway Out12/1 -> Scope2/3
  ? -> Scope2/4
  Gateway Out13/1 -> Scope3/1
  Gateway Out14/1 -> Scope3/2
  Gateway Out15/1 -> Scope3/3
  zero_mask/1(Out1) -> fft_direct2/3(in0)
  zero_mask/2(Out2) -> fft_direct2/4(in1)
  zero_mask/3(Out3) -> fft_direct2/5(in2)
  zero_mask/4(Out4) -> fft_direct2/6(in3)
  [x_zero_mask] -> zero_mask/5(en_mask)
  [y_zero_mask] -> zero_mask1/9(en_mask)
  zero_mask1/1(Out1) -> fft_direct1/3(in0)
  zero_mask1/2(Out2) -> fft_direct1/4(in1)
  zero_mask1/3(Out3) -> fft_direct1/5(in2)
  zero_mask1/4(Out4) -> fft_direct1/6(in3)
  zero_mask1/5(Out5) -> fft_direct1/7(in4)
  zero_mask1/6(Out6) -> fft_direct1/8(in5)
  zero_mask1/7(Out7) -> fft_direct1/9(in6)
  zero_mask1/8(Out8) -> fft_direct1/10(in7)
  Slice5/1 -> [x_zero_mask]
  Slice7/1 -> [y_zero_mask]
  Slice10/1 -> [cnt_rst]
  acc_scale/1(reg_in) -> Slice11/1
  Slice11/1 -> [acc_scale]
  Constant5/1 -> acc_scale/1(sim_reg_in)
  convert_of/1(dout) -> Delay7/1
  convert_of/2(of) -> Delay6/1
  [mini_vacc_of] -> Register2/1, Register2/3
  Gateway Out17/1 -> Scope4/1
  Gateway Out18/1 -> Scope4/2
  Gateway Out19/1 -> Scope4/3
  ? -> Scope4/4
  16_power/1(Out1) -> pow_sim_mux12/2(din)
  pow_sim_mux12/1(dout) -> [Y0_pow]
  16_power/2(Out2) -> pow_sim_mux13/2(din)
  pow_sim_mux13/1(dout) -> [Y1_pow]
  16_power/3(Out3) -> pow_sim_mux14/2(din)
  pow_sim_mux14/1(dout) -> [Y2_pow]
  16_power/4(Out4) -> pow_sim_mux11/2(din)
  pow_sim_mux11/1(dout) -> [Y3_pow]
  16_power/5(Out5) -> pow_sim_mux10/2(din)
  pow_sim_mux10/1(dout) -> [Y4_pow]
  16_power/6(Out6) -> pow_sim_mux9/2(din)
  pow_sim_mux9/1(dout) -> [Y5_pow]
  16_power/7(Out7) -> pow_sim_mux15/2(din)
  pow_sim_mux15/1(dout) -> [Y6_pow]
  16_power/8(Out8) -> pow_sim_mux8/2(din)
  pow_sim_mux8/1(dout) -> [Y7_pow]
  16_power/9(Out9) -> pow_sim_mux7/2(din)
  pow_sim_mux7/1(dout) -> [Y8_pow]
  16_power/10(Out10) -> pow_sim_mux6/2(din)
  pow_sim_mux6/1(dout) -> [Y9_pow]
  16_power/11(Out11) -> pow_sim_mux5/2(din)
  pow_sim_mux5/1(dout) -> [Y10_pow]
  16_power/12(Out12) -> pow_sim_mux4/2(din)
  pow_sim_mux4/1(dout) -> [Y11_pow]
  16_power/13(Out13) -> pow_sim_mux3/2(din)
  pow_sim_mux3/1(dout) -> [Y12_pow]
  16_power/14(Out14) -> pow_sim_mux2/2(din)
  pow_sim_mux2/1(dout) -> [Y13_pow]
  16_power/15(Out15) -> pow_sim_mux1/2(din)
  pow_sim_mux1/1(dout) -> [Y14_pow]
  16_power/16(Out16) -> pow_sim_mux/2(din)
  pow_sim_mux/1(dout) -> [Y15_pow]
  vacc_cnt0/1(sim_reg_out) -> Terminator12/1
  vacc_monitor/2(spec_cnt) -> vacc_cnt0/1(reg_out)
  vacc_err_cnt0/1(sim_reg_out) -> Terminator10/1
  vacc_monitor/1(error_cnt) -> vacc_err_cnt0/1(reg_out)
  [acc_ctr_rst] -> vacc_monitor/1(cnt_rst)
  vacc_mcnt_h0/1(sim_reg_out) -> Terminator13/1
  vacc_mcnt_l0/1(sim_reg_out) -> Terminator33/1
  [new_acc] -> vacc_monitor/3(new_acc)
  [vacc_error] -> vacc_monitor/2(error)
  [window_sync] -> Delay15/1
  [curr_count] -> Register4/1
  [x_fft_sync] -> Register6/2
  Register4/1 -> Register6/1
  [y_fft_sync] -> Register8/2
  Register8/1 -> Register10/1
  [pow_sync_out] -> Register10/2
  Register10/1 -> Register11/1
  [mini_vacc_sync_out] -> Register11/2
  Register11/1 -> Register13/1
  qdr_vacc/2(data_out) -> snap_vacc0/1(din)
  qdr_vacc/4(valid) -> snap_vacc0/2(we)
  snap_vacc0/1(fetch) -> qdr_vacc/6(re)
  qdr_vacc/5(error) -> [vacc_error]
  buffer4/4(mcnt_out) -> [curr_count]
  buffer4/3(window_vld) -> [buf_vld_out], payload_cnt1/2, payload_cnt3/2, bufout_snap/3(we)
  buffer4/1(sync_out) -> [buf_sync_out], payload_cnt1/1, Register0/2, bufout_snap/2(trig)
  buffer4/2(dout) -> [data_buf], bufout_snap/1(din)
  [buf0_data] -> buffer4/1(din)
  [buf0_we] -> buffer4/2(vld)
  [buf0_eof] -> buffer4/3(eof)
  [buf0_mcnt] -> buffer4/4(mcnt)
  [buf0_ant] -> buffer4/6(ant)
  [feng_mrst] -> Delay/1
  Delay/1 -> [buf0_mrst]
  [buf0_mrst] -> buffer4/5(mrst)
  Register6/1 -> Register3/1
  [ct_sync_out] -> Register3/2
  Register3/1 -> Register8/1
  buffer4/5(new_window) -> [window_sync], payload_cnt3/1, Register14/2
  Delay4/1 -> Delay13/1
  Delay5/1 -> Delay12/1
  Delay6/1 -> Delay11/1
  Delay7/1 -> Delay10/1
  Delay10/1 -> [qdr_vacc_data]
  Delay11/1 -> [mini_vacc_of]
  Delay12/1 -> [qdr_vacc_sync]
  Delay13/1 -> [qdr_in_vld]
  sync_gen/1(Out1) -> [qdr_sync], Register5/2, payload_cnt2/1
  Delay14/1 -> [last_mcnt]
  [acc_ctr_rst] -> vacc_monitor2/1(cnt_rst)
  [xaui_pkt_err] -> vacc_monitor2/2(error0)
  vacc_monitor2/1(cnt0) -> xaui_error_cnt0/1(reg_out)
  payload_cnt1/1 -> Register0/1
  Register0/1 -> xauibuf_out_cnt/1(reg_out)
  payload_cnt2/1 -> Register5/1
  Register5/1 -> qdr_in_cnt/1(reg_out)
  [qdr_in_vld] -> payload_cnt2/2
  [acc_ctr_rst] -> vacc_monitor1/1(cnt_rst)
  [xaui_pkt_eof] -> vacc_monitor1/2(error0)
  vacc_monitor1/1(cnt0) -> xaui_cnt0/1(reg_out)
  Slice6/1 -> [status_rst]
  [xaui0_valid] -> Register23/1, Register23/3
  [buf_vld_out] -> Register24/1, Register24/3
  [buf_sync_out] -> Register25/1, Register25/3
  [qdr_sync] -> Register26/1, Register26/3
  [qdr_in_vld] -> Register27/1, Register27/3
  [new_acc] -> Register28/1, Register28/3
  [vacc_error] -> Register29/1, Register29/3
  [status_rst] -> Register29/2, Register28/2, Register27/2, Register26/2, Register25/2, Register24/2, Register23/2, Register2/2, Register1/2, Register/2, Register7/2, Register9/2, Register12/2
  [xaui_pkt_err] -> Register9/1, Register9/3
  [xaui_pkt_eof] -> Register12/1, Register12/3
  [window_sync] -> Register7/1, Register7/3
  Register7/1 -> Concat5/1
  Register/1 -> Concat5/2
  Register1/1 -> Concat5/3
  Register2/1 -> Concat5/4
  Register23/1 -> Concat5/5
  Register24/1 -> Concat5/6
  Register25/1 -> Concat5/7
  Register26/1 -> Concat5/8
  Register27/1 -> Concat5/9
  Register28/1 -> Concat5/10
  Register29/1 -> Concat5/11
  Register9/1 -> Concat5/12
  Register12/1 -> Concat5/13
  payload_cnt3/1 -> Register14/1
  Register14/1 -> xauibuf_window_cnt/1(reg_out)
  Delay15/1 -> Register4/2
  Constant10/1 -> inst_seng_id0/1(reg_out)
  [window_sync] -> x_window/1(sync)
  uncram1/1(Out1) -> x_window/2(In1)
  uncram1/2(Out2) -> x_window/3(In2)
  uncram1/3(Out3) -> x_window/4(In3)
  uncram1/4(Out4) -> x_window/5(In4)
  x_window/1(sync_out) -> fft_direct2/1(sync)
  x_window/2(Out1) -> zero_mask/1(In1)
  x_window/3(Out2) -> zero_mask/2(In2)
  x_window/4(Out3) -> zero_mask/3(In3)
  x_window/5(Out4) -> zero_mask/4(In4)
  [ct_sync_out] -> y_window/1(sync)
  [y0] -> y_window/2(In1)
  [y1] -> y_window/3(In2)
  [y2] -> y_window/4(In3)
  [y3] -> y_window/5(In4)
  [y4] -> y_window/6(In5)
  [y5] -> y_window/7(In6)
  [y6] -> y_window/8(In7)
  [y7] -> y_window/9(In8)
  y_window/1(sync_out) -> fft_direct1/1(sync)
  y_window/2(Out1) -> zero_mask1/1(In1)
  y_window/3(Out2) -> zero_mask1/2(In2)
  y_window/4(Out3) -> zero_mask1/3(In3)
  y_window/5(Out4) -> zero_mask1/4(In4)
  y_window/6(Out5) -> zero_mask1/5(In5)
  y_window/7(Out6) -> zero_mask1/6(In6)
  y_window/8(Out7) -> zero_mask1/7(In7)
  y_window/9(Out8) -> zero_mask1/8(In8)
